simulink model "AcasXuClosedLoop_all_comp"  (Simulink 10.0)
settings: Solver=VariableStepAuto  StartTime=0.0  StopTime=20  RelTol=1e-3
libraries: neural x14040, simulink x2
(part 34 of 534)

== system AcasXuClosedLoop_all_comp/networks/Networks_m_8/AcasXu_3_8/Layer 5 ==
  "a{4} " : Inport  PortDimensions=50
      mask Display: e = 0.06;c1 = [120 230 180]/255;c2 = ([120 230 180]+0)/(256+128);c3 = ([120 230 180]+128)/(256+128);patch([0 1 1 0],[0 0 1 1],c1);patch([0 1 1 1-e 1-e 0+e],[0 0 1 1-e 0+e 0+e],c2);patch([0 0 1 1-e 0+e 0+e],[0 1 1 1-e 1-e 0+e],c3);plot([0 1 1 0 0],[0 0 1 1 0]);
  Delays 1 : Subsystem
      mask Display: e = 0.06;c1 = [120 150 230]/255;c2 = ([120 150 230]+0)/383;c3 = ([120 150 230]+128)/383;patch([0 1 1 0],[0 0 1 1],c1);patch([0 1 1 1-e 1-e 0+e],[0 0 1 1-e 0+e 0+e],c2);patch([0 0 1 1-e 0+e 0+e],[0 1 1 1-e 1-e 0+e],c3);plot([0 1 1 0 0],[0 0 1 1 0]);disp('0');
  "LW{5,4}" : Subsystem
      mask Display: e = 0.06;c1 = [120 150 230]/255;c2 = ([120 150 230]+0)/383;c3 = ([120 150 230]+128)/383;patch([0 1 1 0],[0 0 1 1],c1);patch([0 1 1 1-e 1-e 0+e],[0 0 1 1-e 0+e 0+e],c2);patch([0 0 1 1-e 0+e 0+e],[0 1 1 1-e 1-e 0+e],c3);plot([0 1 1 0 0],[0 0 1 1 0]);disp('W');
  "b{5}" : Constant  Value=[2.504840000000000177493575392873026430606842041015625;2.081119999999999858886212678044103085994720458984375;-2.6239699999999999135980033315718173980712890625;0.005314590000000000112601039603532626642845571041107177734375;-0.1851399999999999990141219541328609921038150787353515625;-0.76549999999999995825561427409411408007144927978515625;-0.0667752000000000067725380858973949216306209564208984375;2.481120000000000214157580558094196021556854248046875;1.084260000000000001563194018672220408916473388671875;1.7157500000000001083577672034152783453464508056640625;0.5807379999999999764526137369102798402309417724609375;-2.84297999999999984055421009543351829051971435546875;2.25241999999999986670218277140520513057708740234375;-2.082120000000000192841298485291190445423126220703125;1.0923599999999999976552089719916693866252899169921875;-1.785649999999999959499064061674289405345916748046875;9.0415299999999998448174665099941194057464599609375;-2.615050000000000096633812063373625278472900390625;1.2832500000000000017763568394002504646778106689453125;-1.3292500000000000426325641456060111522674560546875;-1.4495599999999999596411726088263094425201416015625;0.480439000000000004941824727211496792733669281005859375;-2.525869999999999837569930605241097509860992431640625;-0.065434099999999995045385503544821403920650482177734375;-2.39909999999999978825826474349014461040496826171875;-6.50645000000000006679101716144941747188568115234375;1.8316399999999999348432311307988129556179046630859375;0.353663000000000005140776693224324844777584075927734375;-0.7444610000000000393782784158247523009777069091796875;-0.235028999999999987924326205757097341120243072509765625;3.2953700000000001324451659456826746463775634765625;1.4612899999999999778310666442848742008209228515625;2.267450000000000187583282240666449069976806640625;0.6921370000000000022311041902867145836353302001953125;-0.5316870000000000207052153200493194162845611572265625;-0.9266259999999999497077851629001088440418243408203125;0.886695000000000010942358130705542862415313720703125;-3.08232000000000017081447367672808468341827392578125;-0.362694000000000016381562772949109785258769989013671875;-0.922862999999999988887111612712033092975616455078125;-0.60277700000000000724043047739542089402675628662109375;-2.655130000000000212168060897965915501117706298828125;-0.70483499999999998930633182681049220263957977294921875;0.305122999999999977571718545732437632977962493896484375;0.330502000000000017987389355766936205327510833740234375;-0.4834870000000000000994759830064140260219573974609375;-0.456633000000000011109335673609166406095027923583984375;0.7434849999999999514699311475851573050022125244140625;-0.9466769999999999907203118709730915725231170654296875;3.891480000000000050164317144663073122501373291015625]
      mask Display: e = 0.06;c1 = [120 150 230]/255;c2 = ([120 150 230]+0)/383;c3 = ([120 150 230]+128)/383;patch([0 1 1 0],[0 0 1 1],c1);patch([0 1 1 1-e 1-e 0+e],[0 0 1 1-e 0+e 0+e],c2);patch([0 0 1 1-e 0+e 0+e],[0 1 1 1-e 1-e 0+e],c3);plot([0 1 1 0 0],[0 0 1 1 0]);disp('b');
  netsum : Sum  Inputs=2  SaturateOnIntegerOverflow=on  InputSameDT=on  OutDataTypeStr=Inherit: Same as first input
      mask Display: e = 0.06;c1 = [120 150 230]/255;c2 = ([120 150 230]+0)/383;c3 = ([120 150 230]+128)/383;patch([0 1 1 0],[0 0 1 1],c1);patch([0 1 1 1-e 1-e 0+e],[0 0 1 1-e 0+e 0+e],c2);patch([0 0 1 1-e 0+e 0+e],[0 1 1 1-e 1-e 0+e],c3);plot([0 1 1 0 0],[0 0 1 1 0]);disp('+');
  poslin : neural/Transfer Functions/poslin
      settings: SourceProductName=Deep Learning Toolbox  SourceProductBaseCode=NN
  "a{5}" : Outport  InitialOutput=[0;0;0;0;0;0;0;0;0;0;0;0;0;0;0;0;0;0;0;0;0;0;0;0;0;0;0;0;0;0;0;0;0;0;0;0;0;0;0;0;0;0;0;0;0;0;0;0;0;0]
      mask Display: e = 0.06;c1 = [120 230 180]/255;c2 = ([120 230 180]+0)/(256+128);c3 = ([120 230 180]+128)/(256+128);patch([0 1 1 0],[0 0 1 1],c1);patch([0 1 1 1-e 1-e 0+e],[0 0 1 1-e 0+e 0+e],c2);patch([0 0 1 1-e 0+e 0+e],[0 1 1 1-e 1-e 0+e],c3);plot([0 1 1 0 0],[0 0 1 1 0]);
  wires:
  poslin/1 -> "a{5}"/1
  netsum/1 -> poslin/1
  "b{5}"/1 -> netsum/2
  "LW{5,4}"/1("lz{5,4}") -> netsum/1
  Delays 1/1("pd{5,4}") -> "LW{5,4}"/1("ad{5,4}")
  "a{4} "/1 -> Delays 1/1("p{5}")

== system AcasXuClosedLoop_all_comp/networks/Networks_m_8/AcasXu_3_8/Layer 6 ==
  "a{5} " : Inport  PortDimensions=50
      mask Display: e = 0.06;c1 = [120 230 180]/255;c2 = ([120 230 180]+0)/(256+128);c3 = ([120 230 180]+128)/(256+128);patch([0 1 1 0],[0 0 1 1],c1);patch([0 1 1 1-e 1-e 0+e],[0 0 1 1-e 0+e 0+e],c2);patch([0 0 1 1-e 0+e 0+e],[0 1 1 1-e 1-e 0+e],c3);plot([0 1 1 0 0],[0 0 1 1 0]);
  Delays 1 : Subsystem
      mask Display: e = 0.06;c1 = [120 150 230]/255;c2 = ([120 150 230]+0)/383;c3 = ([120 150 230]+128)/383;patch([0 1 1 0],[0 0 1 1],c1);patch([0 1 1 1-e 1-e 0+e],[0 0 1 1-e 0+e 0+e],c2);patch([0 0 1 1-e 0+e 0+e],[0 1 1 1-e 1-e 0+e],c3);plot([0 1 1 0 0],[0 0 1 1 0]);disp('0');
  "LW{6,5}" : Subsystem
      mask Display: e = 0.06;c1 = [120 150 230]/255;c2 = ([120 150 230]+0)/383;c3 = ([120 150 230]+128)/383;patch([0 1 1 0],[0 0 1 1],c1);patch([0 1 1 1-e 1-e 0+e],[0 0 1 1-e 0+e 0+e],c2);patch([0 0 1 1-e 0+e 0+e],[0 1 1 1-e 1-e 0+e],c3);plot([0 1 1 0 0],[0 0 1 1 0]);disp('W');
  "b{6}" : Constant  Value=[1.1211800000000000654409859635052271187305450439453125;-2.550339999999999829327634870423935353755950927734375;-0.33466600000000001902122903629788197576999664306640625;0.00094879399999999998287225633220032250392250716686248779296875;1.0890400000000000080291329140891321003437042236328125;-8.819610000000000837871994008310139179229736328125;0.641861000000000014864554032101295888423919677734375;-0.035029699999999996951061120853410102427005767822265625;-0.9013240000000000140545353133347816765308380126953125;0.22365299999999999069899558890028856694698333740234375;1.9541299999999999226218960757250897586345672607421875;-7.3797899999999998499333742074668407440185546875;-8.350519999999999498641045647673308849334716796875;0.281984000000000012420287021086551249027252197265625;-3.557420000000000026574298317427746951580047607421875;-1.132130000000000080717654782347381114959716796875;-5.2423400000000004439471013029105961322784423828125;-0.6842740000000000488711293655796907842159271240234375;-1.31238000000000010203393685515038669109344482421875;0.332206999999999974537701064036809839308261871337890625;0.269838999999999995527133478390169329941272735595703125;0.18954999999999999626965063725947402417659759521484375;-10.359899999999999664623828721232712268829345703125;-4.34982999999999986329157763975672423839569091796875;1.43864999999999998436805981327779591083526611328125;-0.347079999999999999626965063725947402417659759521484375;-5.7122200000000002972910806420259177684783935546875;-9.1276399999999995316102285869419574737548828125;1.970050000000000078870243669371120631694793701171875;-6.70408000000000026119550966541282832622528076171875;-6.48481000000000040728309613768942654132843017578125;-0.5031820000000000181472614713129587471485137939453125;0.373782000000000003137046178380842320621013641357421875;2.77784999999999993036681189551018178462982177734375;-6.85794000000000014694023775518871843814849853515625;0.64283999999999996699528992394334636628627777099609375;-1.369429999999999925108795650885440409183502197265625;-0.255975000000000008082423619271139614284038543701171875;0.4617299999999999737809730504523031413555145263671875;-1.02127999999999996560973158921115100383758544921875;0.59191400000000005121592039358802139759063720703125;0.0274540999999999986436183263549537514336407184600830078125;-7.11089999999999999857891452847979962825775146484375;-6.34067000000000025039526008185930550098419189453125;0.8016419999999999657092075722175650298595428466796875;-4.65144000000000001904254531837068498134613037109375;0.334316000000000002057021220025490038096904754638671875;-8.1444799999999997197619450162164866924285888671875;1.619499999999999939603867460391484200954437255859375;-4.37492000000000036408209780347533524036407470703125]
      mask Display: e = 0.06;c1 = [120 150 230]/255;c2 = ([120 150 230]+0)/383;c3 = ([120 150 230]+128)/383;patch([0 1 1 0],[0 0 1 1],c1);patch([0 1 1 1-e 1-e 0+e],[0 0 1 1-e 0+e 0+e],c2);patch([0 0 1 1-e 0+e 0+e],[0 1 1 1-e 1-e 0+e],c3);plot([0 1 1 0 0],[0 0 1 1 0]);disp('b');
  netsum : Sum  Inputs=2  SaturateOnIntegerOverflow=on  InputSameDT=on  OutDataTypeStr=Inherit: Same as first input
      mask Display: e = 0.06;c1 = [120 150 230]/255;c2 = ([120 150 230]+0)/383;c3 = ([120 150 230]+128)/383;patch([0 1 1 0],[0 0 1 1],c1);patch([0 1 1 1-e 1-e 0+e],[0 0 1 1-e 0+e 0+e],c2);patch([0 0 1 1-e 0+e 0+e],[0 1 1 1-e 1-e 0+e],c3);plot([0 1 1 0 0],[0 0 1 1 0]);disp('+');
  poslin : neural/Transfer Functions/poslin
      settings: SourceProductName=Deep Learning Toolbox  SourceProductBaseCode=NN
  "a{6}" : Outport  InitialOutput=[0;0;0;0;0;0;0;0;0;0;0;0;0;0;0;0;0;0;0;0;0;0;0;0;0;0;0;0;0;0;0;0;0;0;0;0;0;0;0;0;0;0;0;0;0;0;0;0;0;0]
      mask Display: e = 0.06;c1 = [120 230 180]/255;c2 = ([120 230 180]+0)/(256+128);c3 = ([120 230 180]+128)/(256+128);patch([0 1 1 0],[0 0 1 1],c1);patch([0 1 1 1-e 1-e 0+e],[0 0 1 1-e 0+e 0+e],c2);patch([0 0 1 1-e 0+e 0+e],[0 1 1 1-e 1-e 0+e],c3);plot([0 1 1 0 0],[0 0 1 1 0]);
  wires:
  poslin/1 -> "a{6}"/1
  netsum/1 -> poslin/1
  "b{6}"/1 -> netsum/2
  "LW{6,5}"/1("lz{6,5}") -> netsum/1
  Delays 1/1("pd{6,5}") -> "LW{6,5}"/1("ad{6,5}")
  "a{5} "/1 -> Delays 1/1("p{6}")

== system AcasXuClosedLoop_all_comp/networks/Networks_m_8/AcasXu_3_8/Layer 7 ==
  "a{6} " : Inport  PortDimensions=50
      mask Display: e = 0.06;c1 = [120 230 180]/255;c2 = ([120 230 180]+0)/(256+128);c3 = ([120 230 180]+128)/(256+128);patch([0 1 1 0],[0 0 1 1],c1);patch([0 1 1 1-e 1-e 0+e],[0 0 1 1-e 0+e 0+e],c2);patch([0 0 1 1-e 0+e 0+e],[0 1 1 1-e 1-e 0+e],c3);plot([0 1 1 0 0],[0 0 1 1 0]);
  Delays 1 : Subsystem
      mask Display: e = 0.06;c1 = [120 150 230]/255;c2 = ([120 150 230]+0)/383;c3 = ([120 150 230]+128)/383;patch([0 1 1 0],[0 0 1 1],c1);patch([0 1 1 1-e 1-e 0+e],[0 0 1 1-e 0+e 0+e],c2);patch([0 0 1 1-e 0+e 0+e],[0 1 1 1-e 1-e 0+e],c3);plot([0 1 1 0 0],[0 0 1 1 0]);disp('0');
  "LW{7,6}" : Subsystem
      mask Display: e = 0.06;c1 = [120 150 230]/255;c2 = ([120 150 230]+0)/383;c3 = ([120 150 230]+128)/383;patch([0 1 1 0],[0 0 1 1],c1);patch([0 1 1 1-e 1-e 0+e],[0 0 1 1-e 0+e 0+e],c2);patch([0 0 1 1-e 0+e 0+e],[0 1 1 1-e 1-e 0+e],c3);plot([0 1 1 0 0],[0 0 1 1 0]);disp('W');
  "b{7}" : Constant  Value=[-0.02092650000000000065636385215839254669845104217529296875;0.0179934999999999990782928449561950401403009891510009765625;-0.019751999999999998725019878520470228977501392364501953125;0.0182035000000000009301448500309561495669186115264892578125;-0.0187597000000000006803002605693109217099845409393310546875]
      mask Display: e = 0.06;c1 = [120 150 230]/255;c2 = ([120 150 230]+0)/383;c3 = ([120 150 230]+128)/383;patch([0 1 1 0],[0 0 1 1],c1);patch([0 1 1 1-e 1-e 0+e],[0 0 1 1-e 0+e 0+e],c2);patch([0 0 1 1-e 0+e 0+e],[0 1 1 1-e 1-e 0+e],c3);plot([0 1 1 0 0],[0 0 1 1 0]);disp('b');
  netsum : Sum  Inputs=2  SaturateOnIntegerOverflow=on  InputSameDT=on  OutDataTypeStr=Inherit: Same as first input
      mask Display: e = 0.06;c1 = [120 150 230]/255;c2 = ([120 150 230]+0)/383;c3 = ([120 150 230]+128)/383;patch([0 1 1 0],[0 0 1 1],c1);patch([0 1 1 1-e 1-e 0+e],[0 0 1 1-e 0+e 0+e],c2);patch([0 0 1 1-e 0+e 0+e],[0 1 1 1-e 1-e 0+e],c3);plot([0 1 1 0 0],[0 0 1 1 0]);disp('+');
  purelin : neural/Transfer Functions/purelin
      settings: SourceProductName=Deep Learning Toolbox
  "a{7}" : Outport  InitialOutput=[0;0;0;0;0]
      mask Display: e = 0.06;c1 = [120 230 180]/255;c2 = ([120 230 180]+0)/(256+128);c3 = ([120 230 180]+128)/(256+128);patch([0 1 1 0],[0 0 1 1],c1);patch([0 1 1 1-e 1-e 0+e],[0 0 1 1-e 0+e 0+e],c2);patch([0 0 1 1-e 0+e 0+e],[0 1 1 1-e 1-e 0+e],c3);plot([0 1 1 0 0],[0 0 1 1 0]);
  wires:
  purelin/1 -> "a{7}"/1
  netsum/1 -> purelin/1
  "b{7}"/1 -> netsum/2
  "LW{7,6}"/1("lz{7,6}") -> netsum/1
  Delays 1/1("pd{7,6}") -> "LW{7,6}"/1("ad{7,6}")
  "a{6} "/1 -> Delays 1/1("p{7}")

== system AcasXuClosedLoop_all_comp/networks/Networks_m_8/AcasXu_3_8/Process Input 1 ==
  x : Inport  PortDimensions=5
      mask Display: e = 0.06;c1 = [120 230 180]/255;c2 = ([120 230 180]+0)/(256+128);c3 = ([120 230 180]+128)/(256+128);patch([0 1 1 0],[0 0 1 1],c1);patch([0 1 1 1-e 1-e 0+e],[0 0 1 1-e 0+e 0+e],c2);patch([0 0 1 1-e 0+e 0+e],[0 1 1 1-e 1-e 0+e],c3);plot([0 1 1 0 0],[0 0 1 1 0]);
  p : Outport  PortDimensions=5
      mask Display: e = 0.06;c1 = [120 230 180]/255;c2 = ([120 230 180]+0)/(256+128);c3 = ([120 230 180]+128)/(256+128);patch([0 1 1 0],[0 0 1 1],c1);patch([0 1 1 1-e 1-e 0+e],[0 0 1 1-e 0+e 0+e],c2);patch([0 0 1 1-e 0+e 0+e],[0 1 1 1-e 1-e 0+e],c3);plot([0 1 1 0 0],[0 0 1 1 0]);
  wires:
  x/1 -> p/1

== system AcasXuClosedLoop_all_comp/networks/Networks_m_8/AcasXu_3_8/Process Output 1 ==
  a : Inport  PortDimensions=5
      mask Display: e = 0.06;c1 = [120 230 180]/255;c2 = ([120 230 180]+0)/(256+128);c3 = ([120 230 180]+128)/(256+128);patch([0 1 1 0],[0 0 1 1],c1);patch([0 1 1 1-e 1-e 0+e],[0 0 1 1-e 0+e 0+e],c2);patch([0 0 1 1-e 0+e 0+e],[0 1 1 1-e 1-e 0+e],c3);plot([0 1 1 0 0],[0 0 1 1 0]);
  y : Outport  PortDimensions=5
      mask Display: e = 0.06;c1 = [120 230 180]/255;c2 = ([120 230 180]+0)/(256+128);c3 = ([120 230 180]+128)/(256+128);patch([0 1 1 0],[0 0 1 1],c1);patch([0 1 1 1-e 1-e 0+e],[0 0 1 1-e 0+e 0+e],c2);patch([0 0 1 1-e 0+e 0+e],[0 1 1 1-e 1-e 0+e],c3);plot([0 1 1 0 0],[0 0 1 1 0]);
  wires:
  a/1 -> y/1

== system AcasXuClosedLoop_all_comp/networks/Networks_m_8/AcasXu_4_8/Layer 1 ==
  "p{1}" : Inport  PortDimensions=5
      mask Display: e = 0.06;c1 = [120 230 180]/255;c2 = ([120 230 180]+0)/(256+128);c3 = ([120 230 180]+128)/(256+128);patch([0 1 1 0],[0 0 1 1],c1);patch([0 1 1 1-e 1-e 0+e],[0 0 1 1-e 0+e 0+e],c2);patch([0 0 1 1-e 0+e 0+e],[0 1 1 1-e 1-e 0+e],c3);plot([0 1 1 0 0],[0 0 1 1 0]);
  Delays 1 : Subsystem
      mask Display: e = 0.06;c1 = [120 150 230]/255;c2 = ([120 150 230]+0)/383;c3 = ([120 150 230]+128)/383;patch([0 1 1 0],[0 0 1 1],c1);patch([0 1 1 1-e 1-e 0+e],[0 0 1 1-e 0+e 0+e],c2);patch([0 0 1 1-e 0+e 0+e],[0 1 1 1-e 1-e 0+e],c3);plot([0 1 1 0 0],[0 0 1 1 0]);disp('0');
  "IW{1,1}" : Subsystem
      mask Display: e = 0.06;c1 = [120 150 230]/255;c2 = ([120 150 230]+0)/383;c3 = ([120 150 230]+128)/383;patch([0 1 1 0],[0 0 1 1],c1);patch([0 1 1 1-e 1-e 0+e],[0 0 1 1-e 0+e 0+e],c2);patch([0 0 1 1-e 0+e 0+e],[0 1 1 1-e 1-e 0+e],c3);plot([0 1 1 0 0],[0 0 1 1 0]);disp('W');
  "b{1}" : Constant  Value=[0.0745408999999999932750682773985317908227443695068359375;-0.0249796000000000011087575302326513337902724742889404296875;-0.32405600000000001070787902790470980107784271240234375;-0.33992699999999997917399241487146355211734771728515625;-0.03289040000000000019131363160340697504580020904541015625;-0.05690420000000000200213179368802229873836040496826171875;-0.14921200000000001129052407122799195349216461181640625;-0.02070249999999999868105504674531402997672557830810546875;0.04931750000000000022648549702353193424642086029052734375;-0.384913000000000005140776693224324844777584075927734375;0.024695399999999999296473873755530803464353084564208984375;-0.29588300000000000711253278495860286056995391845703125;-0.68098300000000000498090457767830230295658111572265625;0.035071999999999999009236972824510303325951099395751953125;0.1644899999999999973265829567026230506598949432373046875;0.194840999999999986425081033303285948932170867919921875;0.017092099999999998960475977582973428070545196533203125;0.491026000000000018008705637839739210903644561767578125;-0.301109000000000015528911490036989562213420867919921875;-0.0172196000000000015717205315013416111469268798828125;-0.1643449999999999910915704504077439196407794952392578125;-0.1629629999999999967474906270581413991749286651611328125;-0.44297100000000000363797880709171295166015625;-0.213029999999999997140065488565596751868724822998046875;0.08083479999999999832116515108282328583300113677978515625;-0.4733870000000000022311041902867145836353302001953125;-0.337002999999999997005062368771177716553211212158203125;-0.1770629999999999981685760985783417709171772003173828125;-0.0713055999999999967631225672448636032640933990478515625;-0.020256799999999998418420688039986998774111270904541015625;-0.1560340000000000060254023992456495761871337890625;-0.36489599999999999813127260495093651115894317626953125;-0.1651060000000000027586821715885889716446399688720703125;-0.283507000000000008999023748401668854057788848876953125;-0.5912800000000000277822209682199172675609588623046875;-0.19400600000000001177369313154486007988452911376953125;-0.0171162000000000015520473795049838372506201267242431640625;0.192560000000000008935074902183259837329387664794921875;-0.0164376000000000001277644656738630146719515323638916015625;-0.1439789999999999958735230620732181705534458160400390625;0.0600918000000000007698730541960685513913631439208984375;-0.2478619999999999989892529583812574855983257293701171875;0.056112799999999997402344575903043732978403568267822265625;0.0114292999999999998761435193728175363503396511077880859375;-0.0274694000000000014549694782317601493559777736663818359375;-0.72131000000000000671462885293294675648212432861328125;-0.08661280000000000367510466503517818637192249298095703125;-0.0230320999999999997953636921010911464691162109375;-0.055846899999999997932231821096138446591794490814208984375;-0.35858299999999998508570797639549709856510162353515625]
      mask Display: e = 0.06;c1 = [120 150 230]/255;c2 = ([120 150 230]+0)/383;c3 = ([120 150 230]+128)/383;patch([0 1 1 0],[0 0 1 1],c1);patch([0 1 1 1-e 1-e 0+e],[0 0 1 1-e 0+e 0+e],c2);patch([0 0 1 1-e 0+e 0+e],[0 1 1 1-e 1-e 0+e],c3);plot([0 1 1 0 0],[0 0 1 1 0]);disp('b');
  netsum : Sum  Inputs=2  SaturateOnIntegerOverflow=on  InputSameDT=on  OutDataTypeStr=Inherit: Same as first input
      mask Display: e = 0.06;c1 = [120 150 230]/255;c2 = ([120 150 230]+0)/383;c3 = ([120 150 230]+128)/383;patch([0 1 1 0],[0 0 1 1],c1);patch([0 1 1 1-e 1-e 0+e],[0 0 1 1-e 0+e 0+e],c2);patch([0 0 1 1-e 0+e 0+e],[0 1 1 1-e 1-e 0+e],c3);plot([0 1 1 0 0],[0 0 1 1 0]);disp('+');
  poslin : neural/Transfer Functions/poslin
      settings: SourceProductName=Deep Learning Toolbox  SourceProductBaseCode=NN
  "a{1}" : Outport  InitialOutput=[0;0;0;0;0;0;0;0;0;0;0;0;0;0;0;0;0;0;0;0;0;0;0;0;0;0;0;0;0;0;0;0;0;0;0;0;0;0;0;0;0;0;0;0;0;0;0;0;0;0]
      mask Display: e = 0.06;c1 = [120 230 180]/255;c2 = ([120 230 180]+0)/(256+128);c3 = ([120 230 180]+128)/(256+128);patch([0 1 1 0],[0 0 1 1],c1);patch([0 1 1 1-e 1-e 0+e],[0 0 1 1-e 0+e 0+e],c2);patch([0 0 1 1-e 0+e 0+e],[0 1 1 1-e 1-e 0+e],c3);plot([0 1 1 0 0],[0 0 1 1 0]);
  wires:
  poslin/1 -> "a{1}"/1
  netsum/1 -> poslin/1
  "b{1}"/1 -> netsum/2
  "IW{1,1}"/1("iz{1,1}") -> netsum/1
  Delays 1/1("pd{1,1}") -> "IW{1,1}"/1("pd{1,1}")
  "p{1}"/1 -> Delays 1/1("p{1}")

== system AcasXuClosedLoop_all_comp/networks/Networks_m_8/AcasXu_4_8/Layer 2 ==
  "a{1} " : Inport  PortDimensions=50
      mask Display: e = 0.06;c1 = [120 230 180]/255;c2 = ([120 230 180]+0)/(256+128);c3 = ([120 230 180]+128)/(256+128);patch([0 1 1 0],[0 0 1 1],c1);patch([0 1 1 1-e 1-e 0+e],[0 0 1 1-e 0+e 0+e],c2);patch([0 0 1 1-e 0+e 0+e],[0 1 1 1-e 1-e 0+e],c3);plot([0 1 1 0 0],[0 0 1 1 0]);
  Delays 1 : Subsystem
      mask Display: e = 0.06;c1 = [120 150 230]/255;c2 = ([120 150 230]+0)/383;c3 = ([120 150 230]+128)/383;patch([0 1 1 0],[0 0 1 1],c1);patch([0 1 1 1-e 1-e 0+e],[0 0 1 1-e 0+e 0+e],c2);patch([0 0 1 1-e 0+e 0+e],[0 1 1 1-e 1-e 0+e],c3);plot([0 1 1 0 0],[0 0 1 1 0]);disp('0');
  "LW{2,1}" : Subsystem
      mask Display: e = 0.06;c1 = [120 150 230]/255;c2 = ([120 150 230]+0)/383;c3 = ([120 150 230]+128)/383;patch([0 1 1 0],[0 0 1 1],c1);patch([0 1 1 1-e 1-e 0+e],[0 0 1 1-e 0+e 0+e],c2);patch([0 0 1 1-e 0+e 0+e],[0 1 1 1-e 1-e 0+e],c3);plot([0 1 1 0 0],[0 0 1 1 0]);disp('W');
  "b{2}" : Constant  Value=[-0.0160072999999999986131538065592394559644162654876708984375;1.138009999999999966036057230667211115360260009765625;-0.050465000000000002911004770567160448990762233734130859375;0.9256919999999999593143229503766633570194244384765625;-0.5186699999999999644018089384189806878566741943359375;-0.362223000000000017184476064358022995293140411376953125;0.60673900000000002830091716532479040324687957763671875;-1.4323200000000000375877107217092998325824737548828125;-0.00888203000000000082725382100079514202661812305450439453125;-1.518170000000000019468870959826745092868804931640625;-0.5692239999999999522373173022060655057430267333984375;-0.94495399999999996065724872096325270831584930419921875;0.377933000000000018925305766970268450677394866943359375;-0.00466537000000000028065993973314107279293239116668701171875;-0.1256259999999999876774126050804625265300273895263671875;-1.230420000000000069206862463033758103847503662109375;0.774899000000000004462208380573429167270660400390625;0.289789999999999992041921359486877918243408203125;-1.84095999999999992979837770690210163593292236328125;-0.140842999999999995974775401919032447040081024169921875;0.1746910000000000129372068613520241342484951019287109375;-0.258987000000000022748025685359607450664043426513671875;0.08538759999999999406039563609738252125680446624755859375;-0.01430449999999999950717199936889301170594990253448486328125;-0.05417070000000000218332019130684784613549709320068359375;1.572200000000000041922021409845910966396331787109375;0.462849000000000010413003792564268223941326141357421875;-0.284210000000000018172130467064562253654003143310546875;1.783840000000000092228447101661004126071929931640625;-0.284135999999999999676703055229154415428638458251953125;0.81781099999999995464605717643280513584613800048828125;0.58529200000000003445421725700725801289081573486328125;-0.344359000000000026187052526438492350280284881591796875;-0.27581800000000000760991269999067299067974090576171875;0.1510740000000000138324907084097503684461116790771484375;0.93615499999999995939248265131027437746524810791015625;-0.8696450000000000013500311979441903531551361083984375;-0.00380258999999999978969267289130584686063230037689208984375;0.62349200000000004617817239704891107976436614990234375;-0.0209745999999999994944932524276737240143120288848876953125;0.9309849999999999514699311475851573050022125244140625;-0.01212839999999999925084370744343686965294182300567626953125;0.56663699999999994627586374917882494628429412841796875;0.76238399999999995060306900995783507823944091796875;-0.00903151999999999947899009811180803808383643627166748046875;-0.7557080000000000463700189357041381299495697021484375;-0.2258960000000000134523503447780967690050601959228515625;-0.28604499999999999371169678852311335504055023193359375;-0.007402200000000000272570854775722182239405810832977294921875;-0.6622280000000000388382659366470761597156524658203125]
      mask Display: e = 0.06;c1 = [120 150 230]/255;c2 = ([120 150 230]+0)/383;c3 = ([120 150 230]+128)/383;patch([0 1 1 0],[0 0 1 1],c1);patch([0 1 1 1-e 1-e 0+e],[0 0 1 1-e 0+e 0+e],c2);patch([0 0 1 1-e 0+e 0+e],[0 1 1 1-e 1-e 0+e],c3);plot([0 1 1 0 0],[0 0 1 1 0]);disp('b');
  netsum : Sum  Inputs=2  SaturateOnIntegerOverflow=on  InputSameDT=on  OutDataTypeStr=Inherit: Same as first input
      mask Display: e = 0.06;c1 = [120 150 230]/255;c2 = ([120 150 230]+0)/383;c3 = ([120 150 230]+128)/383;patch([0 1 1 0],[0 0 1 1],c1);patch([0 1 1 1-e 1-e 0+e],[0 0 1 1-e 0+e 0+e],c2);patch([0 0 1 1-e 0+e 0+e],[0 1 1 1-e 1-e 0+e],c3);plot([0 1 1 0 0],[0 0 1 1 0]);disp('+');
  poslin : neural/Transfer Functions/poslin
      settings: SourceProductName=Deep Learning Toolbox  SourceProductBaseCode=NN
  "a{2}" : Outport  InitialOutput=[0;0;0;0;0;0;0;0;0;0;0;0;0;0;0;0;0;0;0;0;0;0;0;0;0;0;0;0;0;0;0;0;0;0;0;0;0;0;0;0;0;0;0;0;0;0;0;0;0;0]
      mask Display: e = 0.06;c1 = [120 230 180]/255;c2 = ([120 230 180]+0)/(256+128);c3 = ([120 230 180]+128)/(256+128);patch([0 1 1 0],[0 0 1 1],c1);patch([0 1 1 1-e 1-e 0+e],[0 0 1 1-e 0+e 0+e],c2);patch([0 0 1 1-e 0+e 0+e],[0 1 1 1-e 1-e 0+e],c3);plot([0 1 1 0 0],[0 0 1 1 0]);
  wires:
  poslin/1 -> "a{2}"/1
  netsum/1 -> poslin/1
  "b{2}"/1 -> netsum/2
  "LW{2,1}"/1("lz{2,1}") -> netsum/1
  Delays 1/1("pd{2,1}") -> "LW{2,1}"/1("ad{2,1}")
  "a{1} "/1 -> Delays 1/1("p{2}")

== system AcasXuClosedLoop_all_comp/networks/Networks_m_8/AcasXu_4_8/Layer 3 ==
  "a{2} " : Inport  PortDimensions=50
      mask Display: e = 0.06;c1 = [120 230 180]/255;c2 = ([120 230 180]+0)/(256+128);c3 = ([120 230 180]+128)/(256+128);patch([0 1 1 0],[0 0 1 1],c1);patch([0 1 1 1-e 1-e 0+e],[0 0 1 1-e 0+e 0+e],c2);patch([0 0 1 1-e 0+e 0+e],[0 1 1 1-e 1-e 0+e],c3);plot([0 1 1 0 0],[0 0 1 1 0]);
  Delays 1 : Subsystem
      mask Display: e = 0.06;c1 = [120 150 230]/255;c2 = ([120 150 230]+0)/383;c3 = ([120 150 230]+128)/383;patch([0 1 1 0],[0 0 1 1],c1);patch([0 1 1 1-e 1-e 0+e],[0 0 1 1-e 0+e 0+e],c2);patch([0 0 1 1-e 0+e 0+e],[0 1 1 1-e 1-e 0+e],c3);plot([0 1 1 0 0],[0 0 1 1 0]);disp('0');
  "LW{3,2}" : Subsystem
      mask Display: e = 0.06;c1 = [120 150 230]/255;c2 = ([120 150 230]+0)/383;c3 = ([120 150 230]+128)/383;patch([0 1 1 0],[0 0 1 1],c1);patch([0 1 1 1-e 1-e 0+e],[0 0 1 1-e 0+e 0+e],c2);patch([0 0 1 1-e 0+e 0+e],[0 1 1 1-e 1-e 0+e],c3);plot([0 1 1 0 0],[0 0 1 1 0]);disp('W');
  "b{3}" : Constant  Value=[-1.2347799999999999887023705014144070446491241455078125;-0.264469000000000009631406783228158019483089447021484375;0.267762999999999973255171425989829003810882568359375;-0.033293999999999997208011137672656332142651081085205078125;-0.5207289999999999974278352965484373271465301513671875;1.231689999999999951540985421161167323589324951171875;-0.0221464000000000001133759752747209859080612659454345703125;-3.046749999999999847233311811578460037708282470703125;-2.040970000000000172946101884008385241031646728515625;-3.56102000000000007418066161335445940494537353515625;-0.058797200000000000852384829386210185475647449493408203125;-0.564313999999999982293275024858303368091583251953125;0.92120800000000002683009370230138301849365234375;-0.01876730000000000064819261069715139456093311309814453125;-0.57830700000000001548272621221258305013179779052734375;-0.35691499999999998227195874278550036251544952392578125;-0.42090300000000002711431079660542309284210205078125;-0.049942699999999999593836008671132731251418590545654296875;0.230088999999999987977616910939104855060577392578125;0.0145244999999999992168486784294145763851702213287353515625;-1.79882000000000008554934538551606237888336181640625;0.09047929999999999861604038642326486296951770782470703125;-0.6637859999999999871533873374573886394500732421875;-2.022110000000000074038553066202439367771148681640625;-0.52575300000000002587086100902524776756763458251953125;-1.5960199999999999942446038403431884944438934326171875;0.483320000000000027373658895157859660685062408447265625;0.220299999999999995825561427409411408007144927978515625;-0.0173594000000000005246025835958789684809744358062744140625;-0.9277729999999999588311538900597952306270599365234375;-0.436875999999999986567189580455305986106395721435546875;-1.661869999999999958362195684458129107952117919921875;-1.4547600000000000530775423612794838845729827880859375;1.4793499999999999428013097713119350373744964599609375;-2.054419999999999912887460595811717212200164794921875;-0.924476999999999993207211446133442223072052001953125;-0.62970599999999998797051148358150385320186614990234375;-0.76903500000000002412292587905540131032466888427734375;-0.4263879999999999892423829805920831859111785888671875;-1.2865599999999999258903926602215506136417388916015625;-3.067600000000000104449782156734727323055267333984375;0.97468999999999994532373648326029069721698760986328125;0.304346999999999978658848931445390917360782623291015625;-0.6081950000000000411404244005098007619380950927734375;-1.02446999999999999175770426518283784389495849609375;-0.03560950000000000226219043497621896676719188690185546875;-0.391000999999999987455368000155431218445301055908203125;-0.0073182400000000001616395906012257910333573818206787109375;0.91599699999999995014832165907137095928192138671875;1.5526899999999999035793507573544047772884368896484375]
      mask Display: e = 0.06;c1 = [120 150 230]/255;c2 = ([120 150 230]+0)/383;c3 = ([120 150 230]+128)/383;patch([0 1 1 0],[0 0 1 1],c1);patch([0 1 1 1-e 1-e 0+e],[0 0 1 1-e 0+e 0+e],c2);patch([0 0 1 1-e 0+e 0+e],[0 1 1 1-e 1-e 0+e],c3);plot([0 1 1 0 0],[0 0 1 1 0]);disp('b');
  netsum : Sum  Inputs=2  SaturateOnIntegerOverflow=on  InputSameDT=on  OutDataTypeStr=Inherit: Same as first input
      mask Display: e = 0.06;c1 = [120 150 230]/255;c2 = ([120 150 230]+0)/383;c3 = ([120 150 230]+128)/383;patch([0 1 1 0],[0 0 1 1],c1);patch([0 1 1 1-e 1-e 0+e],[0 0 1 1-e 0+e 0+e],c2);patch([0 0 1 1-e 0+e 0+e],[0 1 1 1-e 1-e 0+e],c3);plot([0 1 1 0 0],[0 0 1 1 0]);disp('+');
  poslin : neural/Transfer Functions/poslin
      settings: SourceProductName=Deep Learning Toolbox  SourceProductBaseCode=NN
  "a{3}" : Outport  InitialOutput=[0;0;0;0;0;0;0;0;0;0;0;0;0;0;0;0;0;0;0;0;0;0;0;0;0;0;0;0;0;0;0;0;0;0;0;0;0;0;0;0;0;0;0;0;0;0;0;0;0;0]
      mask Display: e = 0.06;c1 = [120 230 180]/255;c2 = ([120 230 180]+0)/(256+128);c3 = ([120 230 180]+128)/(256+128);patch([0 1 1 0],[0 0 1 1],c1);patch([0 1 1 1-e 1-e 0+e],[0 0 1 1-e 0+e 0+e],c2);patch([0 0 1 1-e 0+e 0+e],[0 1 1 1-e 1-e 0+e],c3);plot([0 1 1 0 0],[0 0 1 1 0]);
  wires:
  poslin/1 -> "a{3}"/1
  netsum/1 -> poslin/1
  "b{3}"/1 -> netsum/2
  "LW{3,2}"/1("lz{3,2}") -> netsum/1
  Delays 1/1("pd{3,2}") -> "LW{3,2}"/1("ad{3,2}")
  "a{2} "/1 -> Delays 1/1("p{3}")

== system AcasXuClosedLoop_all_comp/networks/Networks_m_8/AcasXu_4_8/Layer 4 ==
  "a{3} " : Inport  PortDimensions=50
      mask Display: e = 0.06;c1 = [120 230 180]/255;c2 = ([120 230 180]+0)/(256+128);c3 = ([120 230 180]+128)/(256+128);patch([0 1 1 0],[0 0 1 1],c1);patch([0 1 1 1-e 1-e 0+e],[0 0 1 1-e 0+e 0+e],c2);patch([0 0 1 1-e 0+e 0+e],[0 1 1 1-e 1-e 0+e],c3);plot([0 1 1 0 0],[0 0 1 1 0]);
  Delays 1 : Subsystem
      mask Display: e = 0.06;c1 = [120 150 230]/255;c2 = ([120 150 230]+0)/383;c3 = ([120 150 230]+128)/383;patch([0 1 1 0],[0 0 1 1],c1);patch([0 1 1 1-e 1-e 0+e],[0 0 1 1-e 0+e 0+e],c2);patch([0 0 1 1-e 0+e 0+e],[0 1 1 1-e 1-e 0+e],c3);plot([0 1 1 0 0],[0 0 1 1 0]);disp('0');
  "LW{4,3}" : Subsystem
      mask Display: e = 0.06;c1 = [120 150 230]/255;c2 = ([120 150 230]+0)/383;c3 = ([120 150 230]+128)/383;patch([0 1 1 0],[0 0 1 1],c1);patch([0 1 1 1-e 1-e 0+e],[0 0 1 1-e 0+e 0+e],c2);patch([0 0 1 1-e 0+e 0+e],[0 1 1 1-e 1-e 0+e],c3);plot([0 1 1 0 0],[0 0 1 1 0]);disp('W');
  "b{4}" : Constant  Value=[1.931869999999999976125764078460633754730224609375;-1.3102899999999999547384277320816181600093841552734375;-0.469499999999999972910558199146180413663387298583984375;-0.431815999999999977632114678272046148777008056640625;1.8428199999999999025845909272902645170688629150390625;-2.5650800000000000267164068645797669887542724609375;-0.161302000000000000934363697524531744420528411865234375;0.5857550000000000256505927609396167099475860595703125;0.8547979999999999467519273821380920708179473876953125;2.696219999999999838991016076761297881603240966796875;2.907290000000000151914036905509419739246368408203125;-2.718480000000000007531752999057061970233917236328125;-0.061312800000000000633093577562249265611171722412109375;-2.4989699999999999135980033315718173980712890625;0.4042499999999999982236431605997495353221893310546875;-2.187489999999999934487959762918762862682342529296875;0.0091285299999999992837107498644400038756430149078369140625;0.233331000000000010619061185934697277843952178955078125;0.1270189999999999930224703348358161747455596923828125;-0.03438059999999999727382515857243561185896396636962890625;2.68806000000000011596057447604835033416748046875;0.36355599999999999027977537480182945728302001953125;-2.250030000000000196536120711243711411952972412109375;-0.2459429999999999949533702192638884298503398895263671875;0.7497700000000000475353090223507024347782135009765625;1.4311599999999999877076106713502667844295501708984375;0.87412100000000003685585170387639664113521575927734375;-0.0060245300000000001627853407626389525830745697021484375;-0.52031499999999997196908907426404766738414764404296875;-0.74567499999999997672972540385671891272068023681640625;3.054110000000000102460262496606446802616119384765625;-0.2505319999999999769357827972271479666233062744140625;-0.48413600000000001077893330148071981966495513916015625;1.6635299999999999531752337134093977510929107666015625;0.6072530000000000427462509833276271820068359375;4.43001000000000022538415578310377895832061767578125;-1.1711199999999999388222704510553739964962005615234375;0.352899000000000018228973885925370268523693084716796875;-0.2464659999999999906439285268788808025419712066650390625;-0.7805809999999999693187646698788739740848541259765625;-0.0223485999999999997156496789330049068666994571685791015625;1.6128299999999999858601995583740063011646270751953125;-0.82556499999999999328537114706705324351787567138671875;-1.33635999999999999232613845379091799259185791015625;-0.261263999999999996237676214150269515812397003173828125;-1.6321600000000000552091705685597844421863555908203125;0.041435199999999998421440494666967424564063549041748046875;2.39308999999999993946175891323946416378021240234375;-0.412243999999999999328537114706705324351787567138671875;-1.2925899999999999057109789646347053349018096923828125]
      mask Display: e = 0.06;c1 = [120 150 230]/255;c2 = ([120 150 230]+0)/383;c3 = ([120 150 230]+128)/383;patch([0 1 1 0],[0 0 1 1],c1);patch([0 1 1 1-e 1-e 0+e],[0 0 1 1-e 0+e 0+e],c2);patch([0 0 1 1-e 0+e 0+e],[0 1 1 1-e 1-e 0+e],c3);plot([0 1 1 0 0],[0 0 1 1 0]);disp('b');
  netsum : Sum  Inputs=2  SaturateOnIntegerOverflow=on  InputSameDT=on  OutDataTypeStr=Inherit: Same as first input
      mask Display: e = 0.06;c1 = [120 150 230]/255;c2 = ([120 150 230]+0)/383;c3 = ([120 150 230]+128)/383;patch([0 1 1 0],[0 0 1 1],c1);patch([0 1 1 1-e 1-e 0+e],[0 0 1 1-e 0+e 0+e],c2);patch([0 0 1 1-e 0+e 0+e],[0 1 1 1-e 1-e 0+e],c3);plot([0 1 1 0 0],[0 0 1 1 0]);disp('+');
  poslin : neural/Transfer Functions/poslin
      settings: SourceProductName=Deep Learning Toolbox  SourceProductBaseCode=NN
  "a{4}" : Outport  InitialOutput=[0;0;0;0;0;0;0;0;0;0;0;0;0;0;0;0;0;0;0;0;0;0;0;0;0;0;0;0;0;0;0;0;0;0;0;0;0;0;0;0;0;0;0;0;0;0;0;0;0;0]
      mask Display: e = 0.06;c1 = [120 230 180]/255;c2 = ([120 230 180]+0)/(256+128);c3 = ([120 230 180]+128)/(256+128);patch([0 1 1 0],[0 0 1 1],c1);patch([0 1 1 1-e 1-e 0+e],[0 0 1 1-e 0+e 0+e],c2);patch([0 0 1 1-e 0+e 0+e],[0 1 1 1-e 1-e 0+e],c3);plot([0 1 1 0 0],[0 0 1 1 0]);
  wires:
  poslin/1 -> "a{4}"/1
  netsum/1 -> poslin/1
  "b{4}"/1 -> netsum/2
  "LW{4,3}"/1("lz{4,3}") -> netsum/1
  Delays 1/1("pd{4,3}") -> "LW{4,3}"/1("ad{4,3}")
  "a{3} "/1 -> Delays 1/1("p{4}")

== system AcasXuClosedLoop_all_comp/networks/Networks_m_8/AcasXu_4_8/Layer 5 ==
  "a{4} " : Inport  PortDimensions=50
      mask Display: e = 0.06;c1 = [120 230 180]/255;c2 = ([120 230 180]+0)/(256+128);c3 = ([120 230 180]+128)/(256+128);patch([0 1 1 0],[0 0 1 1],c1);patch([0 1 1 1-e 1-e 0+e],[0 0 1 1-e 0+e 0+e],c2);patch([0 0 1 1-e 0+e 0+e],[0 1 1 1-e 1-e 0+e],c3);plot([0 1 1 0 0],[0 0 1 1 0]);
  Delays 1 : Subsystem
      mask Display: e = 0.06;c1 = [120 150 230]/255;c2 = ([120 150 230]+0)/383;c3 = ([120 150 230]+128)/383;patch([0 1 1 0],[0 0 1 1],c1);patch([0 1 1 1-e 1-e 0+e],[0 0 1 1-e 0+e 0+e],c2);patch([0 0 1 1-e 0+e 0+e],[0 1 1 1-e 1-e 0+e],c3);plot([0 1 1 0 0],[0 0 1 1 0]);disp('0');
  "LW{5,4}" : Subsystem
      mask Display: e = 0.06;c1 = [120 150 230]/255;c2 = ([120 150 230]+0)/383;c3 = ([120 150 230]+128)/383;patch([0 1 1 0],[0 0 1 1],c1);patch([0 1 1 1-e 1-e 0+e],[0 0 1 1-e 0+e 0+e],c2);patch([0 0 1 1-e 0+e 0+e],[0 1 1 1-e 1-e 0+e],c3);plot([0 1 1 0 0],[0 0 1 1 0]);disp('W');
  "b{5}" : Constant  Value=[-0.0197313999999999996337596286366533604450523853302001953125;-0.1745740000000000069491079557337798178195953369140625;1.596270000000000077733375292154960334300994873046875;0.443892000000000008785860927673638798296451568603515625;1.6076200000000000489563944938709028065204620361328125;0.30878899999999998016164681757800281047821044921875;0.297323999999999977195130895779584534466266632080078125;2.875690000000000079438677857979200780391693115234375;1.26855999999999990990318110561929643154144287109375;1.7369099999999999539568307227455079555511474609375;0.8259149999999999547384277320816181600093841552734375;0.98084800000000005315570206221309490501880645751953125;2.092960000000000153619339471333660185337066650390625;2.640969999999999817674734003958292305469512939453125;-2.503130000000000077164941103546880185604095458984375;-1.3715200000000000724043047739542089402675628662109375;1.87162000000000006139089236967265605926513671875;-0.189734000000000013752554650636739097535610198974609375;0.77408299999999996554578274299274198710918426513671875;0.54153799999999996384048017716850154101848602294921875;3.468859999999999832454022907768376171588897705078125;0.80228200000000005065459163233754225075244903564453125;0.448006999999999988570920095298788510262966156005859375;1.5806899999999999284483465089579112827777862548828125;3.6363099999999999312194631784223020076751708984375;-1.5089600000000000790123522165231406688690185546875;1.422239999999999948698814478120766580104827880859375;-0.270500000000000018207657603852567262947559356689453125;-0.0299999999999999988897769753748434595763683319091796875;-0.8876749999999999918287585387588478624820709228515625;2.2304300000000001347189027001149952411651611328125;-2.090590000000000170388148035272024571895599365234375;-0.483435000000000003606004383982508443295955657958984375;3.18761999999999989796606314484961330890655517578125;1.59152000000000004575895218295045197010040283203125;1.336209999999999897823954597697593271732330322265625;-0.00536853999999999977055242794676814810372889041900634765625;-0.90345799999999998330935113699524663388729095458984375;0.49352099999999998747313156854943372309207916259765625;0.471389999999999975699438437004573643207550048828125;-4.7493900000000000005684341886080801486968994140625;2.35470999999999985874410413089208304882049560546875;1.4597100000000000630251406619208864867687225341796875;-2.89463999999999987977616910939104855060577392578125;1.42748000000000008213874025386758148670196533203125;-0.382392000000000009674039347373764030635356903076171875;-0.7572069999999999634354708177852444350719451904296875;-0.00865622999999999913345316571167131769470870494842529296875;-2.7205799999999999982946974341757595539093017578125;-0.8755720000000000169393388205207884311676025390625]
      mask Display: e = 0.06;c1 = [120 150 230]/255;c2 = ([120 150 230]+0)/383;c3 = ([120 150 230]+128)/383;patch([0 1 1 0],[0 0 1 1],c1);patch([0 1 1 1-e 1-e 0+e],[0 0 1 1-e 0+e 0+e],c2);patch([0 0 1 1-e 0+e 0+e],[0 1 1 1-e 1-e 0+e],c3);plot([0 1 1 0 0],[0 0 1 1 0]);disp('b');
  netsum : Sum  Inputs=2  SaturateOnIntegerOverflow=on  InputSameDT=on  OutDataTypeStr=Inherit: Same as first input
      mask Display: e = 0.06;c1 = [120 150 230]/255;c2 = ([120 150 230]+0)/383;c3 = ([120 150 230]+128)/383;patch([0 1 1 0],[0 0 1 1],c1);patch([0 1 1 1-e 1-e 0+e],[0 0 1 1-e 0+e 0+e],c2);patch([0 0 1 1-e 0+e 0+e],[0 1 1 1-e 1-e 0+e],c3);plot([0 1 1 0 0],[0 0 1 1 0]);disp('+');
  poslin : neural/Transfer Functions/poslin
      settings: SourceProductName=Deep Learning Toolbox  SourceProductBaseCode=NN
  "a{5}" : Outport  InitialOutput=[0;0;0;0;0;0;0;0;0;0;0;0;0;0;0;0;0;0;0;0;0;0;0;0;0;0;0;0;0;0;0;0;0;0;0;0;0;0;0;0;0;0;0;0;0;0;0;0;0;0]
      mask Display: e = 0.06;c1 = [120 230 180]/255;c2 = ([120 230 180]+0)/(256+128);c3 = ([120 230 180]+128)/(256+128);patch([0 1 1 0],[0 0 1 1],c1);patch([0 1 1 1-e 1-e 0+e],[0 0 1 1-e 0+e 0+e],c2);patch([0 0 1 1-e 0+e 0+e],[0 1 1 1-e 1-e 0+e],c3);plot([0 1 1 0 0],[0 0 1 1 0]);
  wires:
  poslin/1 -> "a{5}"/1
  netsum/1 -> poslin/1
  "b{5}"/1 -> netsum/2
  "LW{5,4}"/1("lz{5,4}") -> netsum/1
  Delays 1/1("pd{5,4}") -> "LW{5,4}"/1("ad{5,4}")
  "a{4} "/1 -> Delays 1/1("p{5}")

== system AcasXuClosedLoop_all_comp/networks/Networks_m_8/AcasXu_4_8/Layer 6 ==
  "a{5} " : Inport  PortDimensions=50
      mask Display: e = 0.06;c1 = [120 230 180]/255;c2 = ([120 230 180]+0)/(256+128);c3 = ([120 230 180]+128)/(256+128);patch([0 1 1 0],[0 0 1 1],c1);patch([0 1 1 1-e 1-e 0+e],[0 0 1 1-e 0+e 0+e],c2);patch([0 0 1 1-e 0+e 0+e],[0 1 1 1-e 1-e 0+e],c3);plot([0 1 1 0 0],[0 0 1 1 0]);
  Delays 1 : Subsystem
      mask Display: e = 0.06;c1 = [120 150 230]/255;c2 = ([120 150 230]+0)/383;c3 = ([120 150 230]+128)/383;patch([0 1 1 0],[0 0 1 1],c1);patch([0 1 1 1-e 1-e 0+e],[0 0 1 1-e 0+e 0+e],c2);patch([0 0 1 1-e 0+e 0+e],[0 1 1 1-e 1-e 0+e],c3);plot([0 1 1 0 0],[0 0 1 1 0]);disp('0');
  "LW{6,5}" : Subsystem
      mask Display: e = 0.06;c1 = [120 150 230]/255;c2 = ([120 150 230]+0)/383;c3 = ([120 150 230]+128)/383;patch([0 1 1 0],[0 0 1 1],c1);patch([0 1 1 1-e 1-e 0+e],[0 0 1 1-e 0+e 0+e],c2);patch([0 0 1 1-e 0+e 0+e],[0 1 1 1-e 1-e 0+e],c3);plot([0 1 1 0 0],[0 0 1 1 0]);disp('W');
  "b{6}" : Constant  Value=[0.2145269999999999954720664163687615655362606048583984375;-7.86336000000000012732925824820995330810546875;0.053205000000000002291500322826323099434375762939453125;-0.1097930000000000017035262089848401956260204315185546875;1.117529999999999912319026407203637063503265380859375;-4.1026600000000001955413608811795711517333984375;-7.99359000000000019525714378687553107738494873046875;0.036926699999999999801669758880962035618722438812255859375;-6.8559099999999997265831552795134484767913818359375;-5.78938000000000041467274058959446847438812255859375;1.804300000000000014921397450962103903293609619140625;0.57387200000000004873612624578527174890041351318359375;6.32504000000000043968384488834999501705169677734375;-7.19840000000000035385028240852989256381988525390625;-1.06519999999999992468247000942938029766082763671875;-3.77184000000000008157030606525950133800506591796875;-2.2070699999999998652810972998850047588348388671875;0.47399599999999997290700548546737991273403167724609375;-0.906228000000000033509195418446324765682220458984375;-6.294959999999999666897565475665032863616943359375;0.77566199999999996261834667166112922132015228271484375;-3.14271000000000011453948900452814996242523193359375;-7.56409000000000020236257114447653293609619140625;0.67588700000000001555378048578859306871891021728515625;3.836530000000000217852402784046716988086700439453125;-8.6259800000000002029310053330846130847930908203125;-7.00631999999999965922370392945595085620880126953125;0.156494999999999995221600102013326250016689300537109375;-6.91514999999999968594011079403571784496307373046875;0.118611999999999995214494674655725248157978057861328125;1.21914999999999995594635038287378847599029541015625;-0.1865549999999999986055598810708033852279186248779296875;1.1188700000000000311928260998683981597423553466796875;-6.9544300000000003336708687129430472850799560546875;2.55534000000000016683543435647152364253997802734375;-6.9633500000000001506350599811412394046783447265625;-7.04328000000000020719426174764521420001983642578125;-0.92295000000000004813927034774678759276866912841796875;-0.09336899999999999366639968911840696819126605987548828125;-6.5220099999999998630073605454526841640472412109375;-1.336160000000000014352963262354023754596710205078125;-0.9621750000000000024868995751603506505489349365234375;-8.249739999999999184865373536013066768646240234375;-3.30234000000000005314859663485549390316009521484375;-6.65486000000000021969981389702297747135162353515625;-6.3371399999999997731947587453760206699371337890625;6.32763999999999970924591252696700394153594970703125;-9.36683000000000021145751816220581531524658203125;1.2984899999999999220534618871170096099376678466796875;-0.57613400000000003498001888146973215043544769287109375]
      mask Display: e = 0.06;c1 = [120 150 230]/255;c2 = ([120 150 230]+0)/383;c3 = ([120 150 230]+128)/383;patch([0 1 1 0],[0 0 1 1],c1);patch([0 1 1 1-e 1-e 0+e],[0 0 1 1-e 0+e 0+e],c2);patch([0 0 1 1-e 0+e 0+e],[0 1 1 1-e 1-e 0+e],c3);plot([0 1 1 0 0],[0 0 1 1 0]);disp('b');
  netsum : Sum  Inputs=2  SaturateOnIntegerOverflow=on  InputSameDT=on  OutDataTypeStr=Inherit: Same as first input
      mask Display: e = 0.06;c1 = [120 150 230]/255;c2 = ([120 150 230]+0)/383;c3 = ([120 150 230]+128)/383;patch([0 1 1 0],[0 0 1 1],c1);patch([0 1 1 1-e 1-e 0+e],[0 0 1 1-e 0+e 0+e],c2);patch([0 0 1 1-e 0+e 0+e],[0 1 1 1-e 1-e 0+e],c3);plot([0 1 1 0 0],[0 0 1 1 0]);disp('+');
  poslin : neural/Transfer Functions/poslin
      settings: SourceProductName=Deep Learning Toolbox  SourceProductBaseCode=NN
  "a{6}" : Outport  InitialOutput=[0;0;0;0;0;0;0;0;0;0;0;0;0;0;0;0;0;0;0;0;0;0;0;0;0;0;0;0;0;0;0;0;0;0;0;0;0;0;0;0;0;0;0;0;0;0;0;0;0;0]
      mask Display: e = 0.06;c1 = [120 230 180]/255;c2 = ([120 230 180]+0)/(256+128);c3 = ([120 230 180]+128)/(256+128);patch([0 1 1 0],[0 0 1 1],c1);patch([0 1 1 1-e 1-e 0+e],[0 0 1 1-e 0+e 0+e],c2);patch([0 0 1 1-e 0+e 0+e],[0 1 1 1-e 1-e 0+e],c3);plot([0 1 1 0 0],[0 0 1 1 0]);
  wires:
  poslin/1 -> "a{6}"/1
  netsum/1 -> poslin/1
  "b{6}"/1 -> netsum/2
  "LW{6,5}"/1("lz{6,5}") -> netsum/1
  Delays 1/1("pd{6,5}") -> "LW{6,5}"/1("ad{6,5}")
  "a{5} "/1 -> Delays 1/1("p{6}")

== system AcasXuClosedLoop_all_comp/networks/Networks_m_8/AcasXu_4_8/Layer 7 ==
  "a{6} " : Inport  PortDimensions=50
      mask Display: e = 0.06;c1 = [120 230 180]/255;c2 = ([120 230 180]+0)/(256+128);c3 = ([120 230 180]+128)/(256+128);patch([0 1 1 0],[0 0 1 1],c1);patch([0 1 1 1-e 1-e 0+e],[0 0 1 1-e 0+e 0+e],c2);patch([0 0 1 1-e 0+e 0+e],[0 1 1 1-e 1-e 0+e],c3);plot([0 1 1 0 0],[0 0 1 1 0]);
  Delays 1 : Subsystem
      mask Display: e = 0.06;c1 = [120 150 230]/255;c2 = ([120 150 230]+0)/383;c3 = ([120 150 230]+128)/383;patch([0 1 1 0],[0 0 1 1],c1);patch([0 1 1 1-e 1-e 0+e],[0 0 1 1-e 0+e 0+e],c2);patch([0 0 1 1-e 0+e 0+e],[0 1 1 1-e 1-e 0+e],c3);plot([0 1 1 0 0],[0 0 1 1 0]);disp('0');
  "LW{7,6}" : Subsystem
      mask Display: e = 0.06;c1 = [120 150 230]/255;c2 = ([120 150 230]+0)/383;c3 = ([120 150 230]+128)/383;patch([0 1 1 0],[0 0 1 1],c1);patch([0 1 1 1-e 1-e 0+e],[0 0 1 1-e 0+e 0+e],c2);patch([0 0 1 1-e 0+e 0+e],[0 1 1 1-e 1-e 0+e],c3);plot([0 1 1 0 0],[0 0 1 1 0]);disp('W');
  "b{7}" : Constant  Value=[-0.0141948999999999998788968724738879245705902576446533203125;-0.0183209000000000010732748023656313307583332061767578125;0.019646299999999998486810426356896641664206981658935546875;-0.0174695999999999983243181844727587304078042507171630859375;0.0203015000000000001012523398458142764866352081298828125]
      mask Display: e = 0.06;c1 = [120 150 230]/255;c2 = ([120 150 230]+0)/383;c3 = ([120 150 230]+128)/383;patch([0 1 1 0],[0 0 1 1],c1);patch([0 1 1 1-e 1-e 0+e],[0 0 1 1-e 0+e 0+e],c2);patch([0 0 1 1-e 0+e 0+e],[0 1 1 1-e 1-e 0+e],c3);plot([0 1 1 0 0],[0 0 1 1 0]);disp('b');
  netsum : Sum  Inputs=2  SaturateOnIntegerOverflow=on  InputSameDT=on  OutDataTypeStr=Inherit: Same as first input
      mask Display: e = 0.06;c1 = [120 150 230]/255;c2 = ([120 150 230]+0)/383;c3 = ([120 150 230]+128)/383;patch([0 1 1 0],[0 0 1 1],c1);patch([0 1 1 1-e 1-e 0+e],[0 0 1 1-e 0+e 0+e],c2);patch([0 0 1 1-e 0+e 0+e],[0 1 1 1-e 1-e 0+e],c3);plot([0 1 1 0 0],[0 0 1 1 0]);disp('+');
  purelin : neural/Transfer Functions/purelin
      settings: SourceProductName=Deep Learning Toolbox
  "a{7}" : Outport  InitialOutput=[0;0;0;0;0]
      mask Display: e = 0.06;c1 = [120 230 180]/255;c2 = ([120 230 180]+0)/(256+128);c3 = ([120 230 180]+128)/(256+128);patch([0 1 1 0],[0 0 1 1],c1);patch([0 1 1 1-e 1-e 0+e],[0 0 1 1-e 0+e 0+e],c2);patch([0 0 1 1-e 0+e 0+e],[0 1 1 1-e 1-e 0+e],c3);plot([0 1 1 0 0],[0 0 1 1 0]);
  wires:
  purelin/1 -> "a{7}"/1
  netsum/1 -> purelin/1
  "b{7}"/1 -> netsum/2
  "LW{7,6}"/1("lz{7,6}") -> netsum/1
  Delays 1/1("pd{7,6}") -> "LW{7,6}"/1("ad{7,6}")
  "a{6} "/1 -> Delays 1/1("p{7}")

== system AcasXuClosedLoop_all_comp/networks/Networks_m_8/AcasXu_4_8/Process Input 1 ==
  x : Inport  PortDimensions=5
      mask Display: e = 0.06;c1 = [120 230 180]/255;c2 = ([120 230 180]+0)/(256+128);c3 = ([120 230 180]+128)/(256+128);patch([0 1 1 0],[0 0 1 1],c1);patch([0 1 1 1-e 1-e 0+e],[0 0 1 1-e 0+e 0+e],c2);patch([0 0 1 1-e 0+e 0+e],[0 1 1 1-e 1-e 0+e],c3);plot([0 1 1 0 0],[0 0 1 1 0]);
  p : Outport  PortDimensions=5
      mask Display: e = 0.06;c1 = [120 230 180]/255;c2 = ([120 230 180]+0)/(256+128);c3 = ([120 230 180]+128)/(256+128);patch([0 1 1 0],[0 0 1 1],c1);patch([0 1 1 1-e 1-e 0+e],[0 0 1 1-e 0+e 0+e],c2);patch([0 0 1 1-e 0+e 0+e],[0 1 1 1-e 1-e 0+e],c3);plot([0 1 1 0 0],[0 0 1 1 0]);
  wires:
  x/1 -> p/1
